simulink model "TosAeb"  (R2021a)
settings: Solver=FixedStepDiscrete  StartTime=0.0  StopTime=inf  FixedStep=0.01  RelTol=1e-3
undefined symbols (not defined in the file or in its folder): ACCModSts, AccSts1, AEB_ObjectMovingOrientation, AEB_ObjectMovingState, CN_BTCActive, CN_CurrentGearR, CN_CurrentGearR_DHT, CN_PTCActive, CN_VDCActive, CN_VSC_EspOff, CN_VSC_FCW_AEB_Error, CN_VSC_FCW_AEB_NoDispay, CN_VSC_FCW_AEB_PerfmDgdtn, CN_VSC_FCW_AEB_TempUnAvlbl, CN_VSC_VehStandstill, CNaccelAvoidMax, CNaccelAvoidMin, CNbrakePedalPress, CNcomfortStrLeftAccelMax, CNcomfortStrLeftAccelMin, CNcomfortStrRightAccelMax, CNcomfortStrRightAccelMin, CNDrvDoorOpen, CNDrvModeEco, CNDrvModeNorm, CNDrvModeOther, CNDrvModeSport, CNDrvSBRreminder, CNNull, CNobjInvalidID, CNPassDoorOpen, CNPassSBRreminder, CNPEBSAccelNegInf, CNPEBSAccelNull, CNPEBSTimeInf, CNPEBSTimeNull, CNSTOPMAX, CNSTOPNULL, CNsubjWidth, CNSwtOFF, CNSwtON, CNTTCMAX, CNTTCNULL, CNVehConfigAEBCycNone, CNVehConfigAEBNone, CNVehConfigAEBPedNone, CNVehConfigFCWNone, CNVehConfigJANone, CollisionSeverity, FALSE, FCWSnvty, JACycObjCalcInfoDefaultParams, JAPedObjCalcInfoDefaultParams, JAVehObjCalcInfoDefaultParams, latCycObjCalcInfoDefaultParams, latPedObjCalcInfoDefaultParams, longObjCalcInfoDefaultParams, ModSts, movingStatus, ObjectType ... (+1112)
libraries: simulink x3031, AEBSFunctionLib x50, Ta_Library x33, AEBSStateMachineLib x23, Mylibrary x12, simulink_extras x7, ObjSelect_lib x3, dspstat3 x2
(part 4 of 102)

== system TosAeb/FcwAebJa_Step_sys/ALG_AEBSystem/ALG_AEBController/AEB_FuncCondCalcApp ==
  note: Version : 20240729
  ACCModSts : Inport  OutDataTypeStr=Enum: ACCModSts
  LCK_Active : Inport  Port=2  OutDataTypeStr=boolean
  subjInfo : Inport  Port=3  OutDataTypeStr=Bus: BusSubjInfo  BusOutputAsStruct=on
  vehCanInfo : Inport  Port=4  OutDataTypeStr=Bus: BusVehCanInfo  BusOutputAsStruct=on
  vehConfigInfo : Inport  Port=5  OutDataTypeStr=Bus: BusVehConfigInfo  BusOutputAsStruct=on
  vehStateCalcInfo : Inport  Port=6  OutDataTypeStr=Bus: BusVehStateCalcInfo  BusOutputAsStruct=on
  longObjCalcInfo : Inport  Port=7  OutDataTypeStr=Bus: BusLongObjCalcInfo  BusOutputAsStruct=on
  latPedTgtCalcInfo : Inport  Port=8  OutDataTypeStr=Bus: BusLatPedTgtCalcInfo  BusOutputAsStruct=on
  latCycObjCalcInfo : Inport  Port=9  OutDataTypeStr=Bus: BusLatCycTgtCalcInfo  BusOutputAsStruct=on
  JAPedTgtCalcInfo : Inport  Port=10  OutDataTypeStr=Bus: BusAEBJAPedTgtCalcInfo  BusOutputAsStruct=on
  JAVehTgtCalcInfo : Inport  Port=11  OutDataTypeStr=Bus: BusAEBJAVehTgtCalcInfo  BusOutputAsStruct=on
  JaCycTgtCalcInfo : Inport  Port=12  OutDataTypeStr=Bus: BusAEBJACycTgtCalcInfo
  AEBModStsFuncImpArb : Inport  Port=13  OutDataTypeStr=Bus: BusAEBModStsFuncImpArb  BusOutputAsStruct=on
  latFuncActiveStateTime : Inport  Port=14  OutDataTypeStr=Bus: BusLatFuncActiveStateTime  BusOutputAsStruct=on
  Bus Creator : BusCreator  Inputs=14
  Bus Creator2 : BusCreator  Inputs=6  OutDataTypeStr=Bus: BusFuncCondCalcInfo  NonVirtualBus=on
      settings: InheritFromInputs=off
  Constant : Constant  Value=2024072901  OutDataTypeStr=uint32
  CycFunctionCondCalc : Subsystem
  JaCycFunctionCondCalc : Subsystem
  JaPedFunctionCondCalc : Subsystem
  JaVehFunctionCondCalc : Subsystem
  LongFunctionCondCalc : Subsystem
  PedFunctionCondCalc : Subsystem
  Terminator : Terminator
  FuncCondCalcInfo : Outport  OutDataTypeStr=Bus: BusFuncCondCalcInfo  BusOutputAsStruct=on
  wires:
  subjInfo/1 -> Bus Creator/3  "subjInfo"
  JAVehTgtCalcInfo/1 -> Bus Creator/11  "AEBJAVehTgtCalcInfo"
  LCK_Active/1 -> Bus Creator/2  "LCK_Active"
  CycFunctionCondCalc/1(cycFuncSuppAborCond) -> Bus Creator2/3  "cycFuncSuppAborCond"
  latCycObjCalcInfo/1 -> Bus Creator/9  "latCycTgtCalcInfo"
  LongFunctionCondCalc/1(vehFuncSuppAborCond) -> Bus Creator2/1  "vehFuncSuppAborCond"
  Bus Creator/1 -> LongFunctionCondCalc/1(inputSignals), PedFunctionCondCalc/1(inputSignals), JaPedFunctionCondCalc/1(inputSignals), JaCycFunctionCondCalc/1(inputSignals), JaVehFunctionCondCalc/1(inputSignals), CycFunctionCondCalc/1(inputSignals)  "inputSignals"
  JAPedTgtCalcInfo/1 -> Bus Creator/10  "AEBJAPedTgtCalcInfo"
  PedFunctionCondCalc/1(pedFuncSuppAborCond) -> Bus Creator2/2  "pedFuncSuppAborCond"
  latFuncActiveStateTime/1 -> Bus Creator/14  "latFuncActiveStateTime"
  vehStateCalcInfo/1 -> Bus Creator/5  "vehStateCalcInfo"
  Constant/1 -> Terminator/1  "AEB_FUNC_VERSION"
  longObjCalcInfo/1 -> Bus Creator/7  "longObjCalcInfo"
  JaPedFunctionCondCalc/1(AEBJAPedFuncSuppAborCond) -> Bus Creator2/4  "JAPedFuncSuppAborCond"
  vehCanInfo/1 -> Bus Creator/4  "vehCanInfo"
  JaCycFunctionCondCalc/1(JaCycFuncSuppAborCond) -> Bus Creator2/6  "JACycFuncSuppAborCond"
  JaVehFunctionCondCalc/1(AEBJAVehFuncSuppAborCond) -> Bus Creator2/5  "JAVehFuncSuppAborCond"
  JaCycTgtCalcInfo/1 -> Bus Creator/12  "JaCycTgtCalcInfo"
  latPedTgtCalcInfo/1 -> Bus Creator/8  "latPedTgtCalcInfo"
  ACCModSts/1 -> Bus Creator/1  "ACCModSts"
  AEBModStsFuncImpArb/1 -> Bus Creator/13  "AEBModStsFuncImpArb"
  vehConfigInfo/1 -> Bus Creator/6  "vehConfigInfo"
  Bus Creator2/1 -> FuncCondCalcInfo/1

== system TosAeb/FcwAebJa_Step_sys/ALG_AEBSystem/ALG_AEBController/AEB_FuncCondCalcInfo_FUS ==
  longObjCalcInfo : Inport
  subjInfo : Inport  Port=2  OutDataTypeStr=Bus: BusSubjInfo
  FuncCondCalcInfo : Inport  Port=3
  Bus Creator : BusCreator  Inputs=3
  Bus Creator2 : BusCreator  Inputs=6  OutDataTypeStr=Bus: BusFuncCondCalcInfo  NonVirtualBus=on
      settings: InheritFromInputs=off
  CycFunctionCondCalc : Subsystem
  JaCycFunctionCondCalc : Subsystem
  JaPedFunctionCondCalc : Subsystem
  JaVehFunctionCondCalc : Subsystem
  LongFunctionCondCalc : Subsystem
  PedFunctionCondCalc : Subsystem
  FuncCondCalcInfo_FUS : Outport  OutDataTypeStr=Bus: BusFuncCondCalcInfo
  wires:
  CycFunctionCondCalc/1(cycFuncSuppAborCond) -> Bus Creator2/3  "cycFuncSuppAborCond"
  longObjCalcInfo/1 -> Bus Creator/1  "longObjCalcInfo"
  JaCycFunctionCondCalc/1(JaCycFuncSuppAborCond) -> Bus Creator2/6  "JACycFuncSuppAborCond"
  PedFunctionCondCalc/1(pedFuncSuppAborCond) -> Bus Creator2/2  "pedFuncSuppAborCond"
  Bus Creator2/1 -> FuncCondCalcInfo_FUS/1
  Bus Creator/1 -> CycFunctionCondCalc/1(inputSignals), JaPedFunctionCondCalc/1(inputSignals), JaCycFunctionCondCalc/1(inputSignals), JaVehFunctionCondCalc/1(inputSignals), PedFunctionCondCalc/1(inputSignals), LongFunctionCondCalc/1(inputSignals)
  FuncCondCalcInfo/1 -> Bus Creator/3  "FuncCondCalcInfo"
  LongFunctionCondCalc/1(vehFuncSuppAborCond) -> Bus Creator2/1  "vehFuncSuppAborCond"
  JaPedFunctionCondCalc/1(AEBJAPedFuncSuppAborCond) -> Bus Creator2/4  "JAPedFuncSuppAborCond"
  JaVehFunctionCondCalc/1(AEBJAVehFuncSuppAborCond) -> Bus Creator2/5  "JAVehFuncSuppAborCond"
  subjInfo/1 -> Bus Creator/2  "subjInfo"

== system TosAeb/FcwAebJa_Step_sys/ALG_AEBSystem/ALG_AEBController/AEB_FuncImpManagementApp ==
  note: Version : 20211028
  note: Atuo Emergency Barke System Function Implement Management
  vehCanInfo : Inport  OutDataTypeStr=Bus: BusVehCanInfo
  subjVel : Inport  Port=2
  subjLongAccel : Inport  Port=3
  VSC_VehStandstill : Inport  Port=4  OutDataTypeStr=boolean
  longObjCalcInfo : Inport  Port=5  OutDataTypeStr=Bus: BusLongObjCalcInfo
  latPedTgtCalcInfo : Inport  Port=6  OutDataTypeStr=Bus: BusLatPedTgtCalcInfo
  latCycObjCalcInfo : Inport  Port=7  OutDataTypeStr=Bus: BusLatCycTgtCalcInfo
  AEBModStsArb : Inport  Port=8  OutDataTypeStr=Bus: BusAEBModStsArb
  Bus Creator : BusCreator  Inputs=7
  Bus Selector : BusSelector  OutputSignals=FCTB_BrkgReq,FCTB_ABA_Req,FCTB_ABP_Req
  Bus Selector2 : BusSelector  OutputSignals=vehCanInfo.AEBAvailable,longObjCalcInfo.LongObjRawInfo.ObjectMovingState,longObjCalcInfo.LongCollisionAssess.accelAvoidLowLvlBrk,longObjCalcInfo.longObjAbsInfo.axAbsObj,vehCanInfo.AEBBAActive,vehCanInfo.AEBIBActive,vehCanInfo.VehicleStandstill,VSC_VehStandstill,vehCanInfo.ABPAvailable,vehCanInfo.ABAavailable,longObjCalcInfo.LongCollisionAssess.longCollisionSeverity,vehCanInfo.ABPActive,vehCanInfo.ABAactive,vehCanInfo.FCTB_ABA_Level,subjVel,vehCanInfo.AWBactive,longObjCalcInfo.LongObjRawInfo.vx,longObjCalcInfo,latPedTgtCalcInfo,vehCanInfo.FCTB_BrkgReqVal,subjLongAccel
  FuncBlockTimeCalc : Subsystem
  FuncImpArbCalc : Subsystem  TreatAsAtomicUnit=on
  FuncManeuArbCalc : Subsystem
  Signal Conversion1 : SignalConversion  ConversionOutput=Nonvirtual bus  OutDataTypeStr=Bus: BusAEBSFuncOutput  OverrideOpt=off
  Signal Conversion2 : SignalConversion  ConversionOutput=Nonvirtual bus  OutDataTypeStr=Bus: BusFuncManeuAborCond  OverrideOpt=off
  AEBSFuncOut : Outport  OutDataTypeStr=Bus: BusAEBSFuncOutput
  FuncBlockTime : Outport  Port=2  OutDataTypeStr=Bus: BusFuncBlockTime
  AEBModStsFuncImpArb : Outport  Port=3  OutDataTypeStr=Bus: BusAEBModStsFuncImpArb
  FuncImpSuppAbor : Outport  Port=4  OutDataTypeStr=Bus: BusFuncImpSuppAborCond
  FuncManeuAborCond : Outport  Port=5  OutDataTypeStr=Bus: BusFuncManeuAborCond
  wires:
  Signal Conversion1/1 -> AEBSFuncOut/1
  Bus Selector2/2 -> FuncManeuArbCalc/3(ObjectMovingState)  "<ObjectMovingState>"
  Bus Selector/2 -> FuncImpArbCalc/3(FCTB_ABAModStsArb)  "<FCTB_ABA_Req>"
  Bus Selector2/20 -> FuncManeuArbCalc/21(FCTB_BrkgReqVal)  "<FCTB_BrkgReqVal>"
  vehCanInfo/1 -> Bus Creator/1, Bus Selector/1  "vehCanInfo"
  FuncBlockTimeCalc/1(funcBlockTime) -> FuncBlockTime/1
  subjVel/1 -> Bus Creator/3  "subjVel"
  Bus Selector2/14 -> FuncManeuArbCalc/15(FCTB_ABA_Level)  "<FCTB_ABA_Level>"
  AEBModStsArb/1 -> FuncImpArbCalc/1(AEBModStsArb)
  subjLongAccel/1 -> Bus Creator/6  "subjLongAccel"
  Bus Selector2/10 -> FuncManeuArbCalc/11(ABAAvailable)  "<ABAavailable>"
  Bus Selector2/12 -> FuncManeuArbCalc/13(ABPActive)  "<ABPActive>"
  VSC_VehStandstill/1 -> Bus Creator/7  "VSC_VehStandstill"
  Bus Selector2/8 -> FuncManeuArbCalc/9(VSC_VehStandstill)  "<VSC_VehStandstill>"
  Bus Selector2/17 -> FuncManeuArbCalc/18(vx)  "<vx>"
  FuncManeuArbCalc/1(AEBSFuncOutput) -> Signal Conversion1/1
  [AEBModStsFuncImpArb] -> AEBModStsFuncImpArb/1
  Bus Selector2/5 -> FuncManeuArbCalc/6(AEBBA)  "<AEBBAActive>"
  FuncManeuArbCalc/2(funcManeuAborCond) -> Signal Conversion2/1
  Bus Selector2/7 -> FuncManeuArbCalc/8(VehicleStandstill)  "<VehicleStandstill>"
  Signal Conversion2/1 -> FuncManeuAborCond/1
  Bus Selector2/3 -> FuncManeuArbCalc/4(accelAvoid)  "<accelAvoidLowLvlBrk>"
  Bus Selector/3 -> FuncImpArbCalc/4(FCTB_PrefillModStsArb)  "<FCTB_ABP_Req>"
  Bus Selector2/9 -> FuncManeuArbCalc/10(ABPAvailable)  "<ABPAvailable>"
  Bus Selector2/4 -> FuncManeuArbCalc/5(axAbsObj)  "<axAbsObj>"
  Bus Selector2/15 -> FuncManeuArbCalc/16(subjVel)  "<subjVel>"
  [funcImpSuppAbor] -> FuncImpSuppAbor/1
  Bus Selector2/11 -> FuncManeuArbCalc/12(longCollisionSeverity)  "<longCollisionSeverity>"
  latCycObjCalcInfo/1 -> Bus Creator/5  "latCycObjCalcInfo"
  Bus Creator/1 -> Bus Selector2/1
  longObjCalcInfo/1 -> Bus Creator/2  "longObjCalcInfo"
  Bus Selector2/6 -> FuncManeuArbCalc/7(AEBIB)  "<AEBIBActive>"
  Bus Selector2/18 -> FuncManeuArbCalc/19(longObjCalcInfo)  "<longObjCalcInfo>"
  Bus Selector/1 -> FuncImpArbCalc/2(FCTBModStsArb)  "<FCTB_BrkgReq>"
  FuncImpArbCalc/2(FuncImpSuppCondAbor) -> [funcImpSuppAbor]
  Bus Selector2/1 -> FuncManeuArbCalc/2(AEBAvailable)  "<AEBAvailable>"
  Bus Selector2/13 -> FuncManeuArbCalc/14(ABAActive)  "<ABAactive>"
  latPedTgtCalcInfo/1 -> Bus Creator/4  "latPedTgtCalcInfo"
  Bus Selector2/16 -> FuncManeuArbCalc/17(AWBActive)  "<AWBactive>"
  Bus Selector2/19 -> FuncManeuArbCalc/20(latPedTgtCalcInfo)  "<latPedTgtCalcInfo>"
  FuncImpArbCalc/1(AEBModStsFuncImpArb) -> [AEBModStsFuncImpArb], FuncManeuArbCalc/1(AEBModStsFuncImpArb), FuncBlockTimeCalc/1(AEBModStsFuncImpArb)
  Bus Selector2/21 -> FuncManeuArbCalc/22(subjLongAccel)  "<subjLongAccel>"

== system TosAeb/FcwAebJa_Step_sys/ALG_AEBSystem/ALG_AEBController/AEB_JaObjCalcApp ==
  JaObjInfo : Inport  OutDataTypeStr=Bus: BusAEBJAObjBus  PortDimensions=32  BusOutputAsStruct=on
  subjInfo : Inport  Port=2  OutDataTypeStr=Bus: BusSubjInfo
  SteerWheelAngle_Deg : Inport  Port=3
  JAPedPrefillModSts : Inport  Port=4  OutDataTypeStr=Enum: ModSts
  JAPedAEBfullModSts : Inport  Port=5  OutDataTypeStr=Enum: ModSts
  JAVehPrefillModSts : Inport  Port=6  OutDataTypeStr=Enum: ModSts
  JAVehAEBfullModSts : Inport  Port=7  OutDataTypeStr=Enum: ModSts
  JaCycPrefillModSts : Inport  Port=8  OutDataTypeStr=Enum: ModSts
  JaCycEBfullModSts : Inport  Port=9  OutDataTypeStr=Enum: ModSts
  Bus Creator : BusCreator  Inputs=9
  Bus Selector : BusSelector  OutputSignals=JaObjInfo,subjInfo,SteerWheelAngle_Deg,JAPedPrefillModSts,JAPedAEBfullModSts
  Bus Selector1 : BusSelector  OutputSignals=JaObjInfo,subjInfo,SteerWheelAngle_Deg,JAVehPrefillModSts,JAVehAEBfullModSts
  Bus Selector2 : BusSelector  OutputSignals=JaObjInfo,subjInfo,SteerWheelAngle_Deg,JaCycPrefillModSts,JaCycEBfullModSts
  JAPedTgtInfoCalc : Subsystem
  JAVehTgtInfoCalc : Subsystem
  JaCycTgtInfoCalc : Subsystem
  JaPedTgtCalcInfo : Outport  OutDataTypeStr=Bus: BusAEBJAPedTgtCalcInfo
  JaVehTgtCalcInfo : Outport  Port=2  OutDataTypeStr=Bus: BusAEBJAVehTgtCalcInfo
  JaCycTgtCalcInfo : Outport  Port=3  OutDataTypeStr=Bus: BusAEBJACycTgtCalcInfo
  wires:
  Bus Selector1/3 -> JAVehTgtInfoCalc/3(SteerWheelAngle_Deg)  "<SteerWheelAngle_Deg>"
  Bus Selector1/4 -> JAVehTgtInfoCalc/4(JAVehPrefillModSts)  "<JAVehPrefillModSts>"
  Bus Selector/4 -> JAPedTgtInfoCalc/4(JAPedPrefillModSts)  "<JAPedPrefillModSts>"
  JaCycEBfullModSts/1 -> Bus Creator/9  "JaCycEBfullModSts"
  Bus Selector/2 -> JAPedTgtInfoCalc/2(subjInfo)  "<subjInfo>"
  Bus Selector2/1 -> JaCycTgtInfoCalc/1(JaObj)  "<JaObjInfo>"
  Bus Selector2/5 -> JaCycTgtInfoCalc/5(JaCycAEBfullModSts)  "<JaCycEBfullModSts>"
  Bus Selector/1 -> JAPedTgtInfoCalc/1(AEBJAObj)  "<JaObjInfo>"
  JAPedPrefillModSts/1 -> Bus Creator/4  "JAPedPrefillModSts"
  Bus Selector2/4 -> JaCycTgtInfoCalc/4(JaCycPrefillModSts)  "<JaCycPrefillModSts>"
  Bus Selector2/3 -> JaCycTgtInfoCalc/3(SteerWheelAngle_Deg)  "<SteerWheelAngle_Deg>"
  Bus Selector1/2 -> JAVehTgtInfoCalc/2(subjInfo)  "<subjInfo>"
  Bus Selector/3 -> JAPedTgtInfoCalc/3(SteerWheelAngle_Deg)  "<SteerWheelAngle_Deg>"
  Bus Selector1/5 -> JAVehTgtInfoCalc/5(JAVehAEBfullModSts)  "<JAVehAEBfullModSts>"
  JaCycPrefillModSts/1 -> Bus Creator/8  "JaCycPrefillModSts"
  subjInfo/1 -> Bus Creator/2  "subjInfo"
  Bus Selector/5 -> JAPedTgtInfoCalc/5(JAPedAEBfullModSts)  "<JAPedAEBfullModSts>"
  SteerWheelAngle_Deg/1 -> Bus Creator/3  "SteerWheelAngle_Deg"
  JAPedTgtInfoCalc/1(AEBJAPedTgtCalcInfo) -> JaPedTgtCalcInfo/1
  JAVehAEBfullModSts/1 -> Bus Creator/7  "JAVehAEBfullModSts"
  JaCycTgtInfoCalc/1(JaCycTgtCalcInfo) -> JaCycTgtCalcInfo/1
  JAPedAEBfullModSts/1 -> Bus Creator/5  "JAPedAEBfullModSts"
  Bus Selector1/1 -> JAVehTgtInfoCalc/1(AEBJAObj)  "<JaObjInfo>"
  JaObjInfo/1 -> Bus Creator/1  "JaObjInfo"
  Bus Selector2/2 -> JaCycTgtInfoCalc/2(subjInfo)  "<subjInfo>"
  JAVehPrefillModSts/1 -> Bus Creator/6  "JAVehPrefillModSts"
  Bus Creator/1 -> Bus Selector2/1, Bus Selector1/1, Bus Selector/1
  JAVehTgtInfoCalc/1(JAVehTgtCalcInfo) -> JaVehTgtCalcInfo/1

== system TosAeb/FcwAebJa_Step_sys/ALG_AEBSystem/ALG_AEBController/AEB_LatObjInfoCalcApp ==
  note: Version : 20240729
  note: 1. 更新LatPedTgt终选条件，增加搜索目标ID 20221011
  FcwHMISetting : Inport  OutDataTypeStr=Enum: FCWSnvty
  latObjInfo : Inport  Port=2  OutDataTypeStr=Bus: BusAEBLatObj  PortDimensions=10  BusOutputAsStruct=on
  subjInfo : Inport  Port=3  OutDataTypeStr=Bus: BusSubjInfo
  LatCycObjCalc : Subsystem
  LatPedObjCalc : Subsystem
  latPedTgtCalcInfo : Outport  OutDataTypeStr=Bus: BusLatPedTgtCalcInfo
  latPedCalcInfo : Outport  Port=2  OutDataTypeStr=Bus: BusLatPedObjCalcInfo
  latCycTgtCalcInfo : Outport  Port=3  OutDataTypeStr=Bus: BusLatCycTgtCalcInfo
  latCycCalcInfo : Outport  Port=4  OutDataTypeStr=Bus: BusLatCycObjCalcInfo
  wires:
  LatPedObjCalc/1(latPedTgtCalcInfo) -> latPedTgtCalcInfo/1
  LatPedObjCalc/2(latPedCalcInfo) -> latPedCalcInfo/1
  LatCycObjCalc/1(latCycTgtCalcInfo) -> latCycTgtCalcInfo/1
  latObjInfo/1 -> LatCycObjCalc/1(latCycObjInfo), LatPedObjCalc/1(latPedObjInfo)  "latPedObjInfo"
  FcwHMISetting/1 -> LatPedObjCalc/3(FcwHMISetting)
  subjInfo/1 -> LatCycObjCalc/2(subjInfo), LatPedObjCalc/2(subjInfo)  "subjInfo"
  LatCycObjCalc/2(latCycCalcInfo) -> latCycCalcInfo/1

== system TosAeb/FcwAebJa_Step_sys/ALG_AEBSystem/ALG_AEBController/AEB_LongTgtInfoCalc ==
  note: AEBS Longitude Function Object Information Calculation
  note: 预测避免碰撞减速度Warning与Brake参数阈值分开处理 20240729
  note: Version : 20240729
  longObjInfo : Inport  OutDataTypeStr=Bus: BusAEBLongObj
  subjInfo : Inport  Port=2  OutDataTypeStr=Bus: BusSubjInfo
  ACCState : Inport  Port=3  OutDataTypeStr=Enum: ACCModSts
  vehCanInfo : Inport  Port=4  OutDataTypeStr=Bus: BusVehCanInfo
  FcwHMISetting : Inport  Port=5  OutDataTypeStr=Enum: FCWSnvty
  Bus Selector5 : BusSelector  OutputSignals=ObjectID
  If : If  IfExpression=u1
  LongObjCalcInfoCalc : Subsystem
  Merge : Merge
  NoTarget : Subsystem  TreatAsAtomicUnit=on
  ObjISInvalid : Subsystem
  longObjCalcInfo : Outport  OutDataTypeStr=Bus: BusLongObjCalcInfo
  wires:
  vehCanInfo/1 -> LongObjCalcInfoCalc/4(vehCanInfo)
  FcwHMISetting/1 -> LongObjCalcInfoCalc/5(FcwHMISetting)
  Bus Selector5/1 -> ObjISInvalid/1(ObjectID)  "<ObjectID>"
  If/2 -> LongObjCalcInfoCalc/Action
  Merge/1 -> longObjCalcInfo/1
  subjInfo/1 -> LongObjCalcInfoCalc/2(subjInfo)
  ACCState/1 -> LongObjCalcInfoCalc/3(ACCState)
  NoTarget/1(longObjCalcInfo) -> Merge/1
  ObjISInvalid/1(ObjIsInvalidFlg) -> If/1
  If/1 -> NoTarget/Action
  LongObjCalcInfoCalc/1(longObjCalcInfo) -> Merge/2
  longObjInfo/1 -> LongObjCalcInfoCalc/1(longObjInfo), Bus Selector5/1

== system TosAeb/FcwAebJa_Step_sys/ALG_AEBSystem/ALG_AEBController/AEB_StateMachineCalcApp ==
  note: AEB State Machine App
  note: Version : 20210816
  AVHStsActv : Inport
  vehStateCalcInfo : Inport  Port=2  OutDataTypeStr=Bus: BusVehStateCalcInfo
  FuncCondCalcInfo : Inport  Port=3  OutDataTypeStr=Bus: BusFuncCondCalcInfo
  FuncImpSuppAborCond : Inport  Port=4  OutDataTypeStr=Bus: BusFuncImpSuppAborCond
  FuncManeuAborCond : Inport  Port=5  OutDataTypeStr=Bus: BusFuncManeuAborCond
  Bus Creator : BusCreator  Inputs=6
  Bus Creator1 : BusCreator  Inputs=5
  Bus Creator2 : BusCreator  Inputs=7
  Bus Creator3 : BusCreator  Inputs=8
  Bus Creator4 : BusCreator  Inputs=3  OutDataTypeStr=Bus: BusAEBModStsArb  NonVirtualBus=on
      settings: InheritFromInputs=off
  Bus Creator5 : BusCreator  Inputs=3  OutDataTypeStr=Bus: BusAEBModRawSts  NonVirtualBus=on
      settings: InheritFromInputs=off
  Bus Selector : BusSelector  OutputSignals=AVHStsActv,vehStateCalcInfo,FuncCondCalcInfo.PedFuncSuppAborCond,FuncCondCalcInfo.CycFuncSuppAborCond,FuncImpSuppAborCond,FuncManeuAborCond
  Bus Selector1 : BusSelector  OutputSignals=AVHStsActv,vehStateCalcInfo,FuncCondCalcInfo.VehFuncSuppAborCond,FuncImpSuppAborCond,FuncManeuAborCond
  Bus Selector2 : BusSelector  OutputSignals=AVHStsActv,vehStateCalcInfo,FuncCondCalcInfo.JAPedFuncSuppAborCond,FuncCondCalcInfo.JAVehFuncSuppAborCond,FuncCondCalcInfo.JACycFuncSuppAborCond,FuncImpSuppAborCond,FuncManeuAborCond
  JA_AEBStateMachine : Subsystem
  JaFctStsArb : Subsystem
  LatAEBStateMachine : Subsystem
  LatFctStsArb : Subsystem
  Unit Delay : UnitDelay  InitialCondition=FALSE  SampleTime=-1  HasFrameUpgradeWarning=on
  Unit Delay1 : UnitDelay  InitialCondition=FALSE  SampleTime=-1  HasFrameUpgradeWarning=on
  Unit Delay2 : UnitDelay  InitialCondition=FALSE  SampleTime=-1  HasFrameUpgradeWarning=on
  VehAEBStateMachine : Subsystem
  VehFCTModStsArb : Subsystem
  AEBModStsArb : Outport  OutDataTypeStr=Bus: BusAEBModStsArb
  latActiveStateTime : Outport  Port=2  OutDataTypeStr=Bus: BusLatFuncActiveStateTime
  AEBModRawSts : Outport  Port=3  OutDataTypeStr=Bus: BusAEBModRawSts
  wires:
  Bus Selector2/4 -> Bus Creator3/4  "<JAVehFuncSuppAborCond>"
  VehFCTModStsArb/1(vehAEBModStsArb) -> Bus Creator4/1  "vehAEBModStsArb"
  JA_AEBStateMachine/1(JA_AEBRawModSts) -> [JA_AEBRawModSts]
  Unit Delay2/1 -> Bus Creator3/8  "JA_FctStsArbSuppAborCond"
  Bus Selector1/2 -> Bus Creator/2  "<vehStateCalcInfo>"
  Bus Selector/3 -> Bus Creator2/3  "<PedFuncSuppAborCond>"
  Bus Selector/6 -> Bus Creator2/6  "<FuncManeuAborCond>"
  vehStateCalcInfo/1 -> Bus Creator1/2  "vehStateCalcInfo"
  LatFctStsArb/1(latAEBModStsArb) -> Bus Creator4/2  "latAEBModStsArb"
  JA_AEBStateMachine/2(JA_AEBModSts) -> JaFctStsArb/1(JA_AEBModSts)
  Bus Selector/4 -> Bus Creator2/4  "<CycFuncSuppAborCond>"
  Bus Creator3/1 -> JA_AEBStateMachine/1(InputSignal)
  Bus Creator4/1 -> AEBModStsArb/1
  Unit Delay1/1 -> Bus Creator2/7  "latFctStsArbSuppAborCond"
  Bus Selector2/5 -> Bus Creator3/5  "<JACycFuncSuppAborCond>"
  Bus Selector1/5 -> Bus Creator/5  "<FuncManeuAborCond>"
  Bus Selector2/7 -> Bus Creator3/7  "<FuncManeuAborCond>"
  Bus Creator/1 -> VehAEBStateMachine/1(InputSignal)
  LatAEBStateMachine/1(latAEBRawModSts) -> [latAEBRawModSts]
  Bus Creator5/1 -> AEBModRawSts/1
  LatAEBStateMachine/3(latActiveStateTime) -> [latActiveStateTime]  "latActiveStateTime"
  [JA_AEBRawModSts] -> Bus Creator5/3  "JA_AEBRawModSts"
  VehAEBStateMachine/2(vehAEBModSts) -> VehFCTModStsArb/1(vehAEBModSts)
  Bus Selector2/3 -> Bus Creator3/3  "<JAPedFuncSuppAborCond>"
  VehAEBStateMachine/1(vehRawModSts) -> [vehRawModSts]
  Bus Selector1/1 -> Bus Creator/1  "<AVHStsActv>"
  [latAEBRawModSts] -> Bus Creator5/2  "latAEBRawModSts"
  LatFctStsArb/2(latFctStsArbSuppAborCond) -> Unit Delay1/1
  [latActiveStateTime] -> latActiveStateTime/1  "latActiveStateTime"
  FuncCondCalcInfo/1 -> Bus Creator1/3  "FuncCondCalcInfo"
  JaFctStsArb/2(vehFctStsArbSuppAborCond) -> Unit Delay2/1
  LatAEBStateMachine/2(latAEBModSts) -> LatFctStsArb/1(latAEBModSts)
  VehFCTModStsArb/2(vehFctStsArbSuppAborCond) -> Unit Delay/1
  Bus Selector1/4 -> Bus Creator/4  "<FuncImpSuppAborCond>"
  [vehRawModSts] -> Bus Creator5/1  "vehRawModSts"
  Bus Selector2/2 -> Bus Creator3/2  "<vehStateCalcInfo>"
  FuncImpSuppAborCond/1 -> Bus Creator1/4  "FuncImpSuppAborCond"
  Bus Creator1/1 -> Bus Selector2/1, Bus Selector/1, Bus Selector1/1
  Bus Selector1/3 -> Bus Creator/3  "<VehFuncSuppAborCond>"
  AVHStsActv/1 -> Bus Creator1/1  "AVHStsActv"
  Bus Selector2/6 -> Bus Creator3/6  "<FuncImpSuppAborCond>"
  Bus Selector/1 -> Bus Creator2/1  "<AVHStsActv>"
  Bus Selector/2 -> Bus Creator2/2  "<vehStateCalcInfo>"
  FuncManeuAborCond/1 -> Bus Creator1/5  "FuncManeuAborCond"
  Bus Selector2/1 -> Bus Creator3/1  "<AVHStsActv>"
  Bus Selector/5 -> Bus Creator2/5  "<FuncImpSuppAborCond>"
  Bus Creator2/1 -> LatAEBStateMachine/1(InputSignal)
  JaFctStsArb/1(JA_AEBModStsArb) -> Bus Creator4/3  "JA_pedAEBModStsArb"
  Unit Delay/1 -> Bus Creator/6  "vehFctStsArbSuppAborCond"

== system TosAeb/FcwAebJa_Step_sys/ALG_AEBSystem/ALG_AEBController/AEB_VehicleStateCalcApp ==
  note: Auto Emergency Brake System Vehicle State Calculation
  note: Version : 20210723
  diagManageInfo : Inport  OutDataTypeStr=Bus: BusDiagManageInfo
  vehCanInfo : Inport  Port=2  OutDataTypeStr=Bus: BusVehCanInfo
  vehConfigInfo : Inport  Port=3  OutDataTypeStr=Bus: BusVehConfigInfo
  subjVel : Inport  Port=4
  funcBlockTime : Inport  Port=5  OutDataTypeStr=Bus: BusFuncBlockTime
  Bus Creator : BusCreator  Inputs=4
  Bus Creator1 : BusCreator  Inputs=5
  Bus Creator2 : BusCreator  Inputs=3  OutDataTypeStr=Bus: BusVSC_FCW_AEB_FctSts
      settings: InheritFromInputs=off
  Bus Creator3 : BusCreator  Inputs=4  OutDataTypeStr=Bus: BusAEBFuncSwtSts
      settings: InheritFromInputs=off
  Bus Creator4 : BusCreator  Inputs=2
  Bus Creator6 : BusCreator  Inputs=7  OutDataTypeStr=Bus: BusVehStateCalcInfo  NonVirtualBus=on
      settings: InheritFromInputs=off
  Bus Selector : BusSelector  OutputSignals=diaManageInfo.FCW_AEB_ErrorState,diaManageInfo.AEB_PEDP_ErrorState,diaManageInfo.AEB_JA_ErrorState,vehCanInfo.EspFuncOffSts,vehCanInfo.TrailerSts
  Bus Selector1 : BusSelector  OutputSignals=vehCanInfo.AEB_VehSwtReq,vehCanInfo.AEB_PedSwtReq,vehCanInfo.AEB_JASwtReq,vehCanInfo.FCW_SnvtySet
  Bus Selector2 : BusSelector  OutputSignals=vehCanInfo.VDCActive,vehCanInfo.BTCActive,vehCanInfo.PTCActive,vehCanInfo.CURRENT_GEAR,vehCanInfo.HCU_GearSts,vehCanInfo.EspFuncOffSts,vehCanInfo.DrivingModeDis,vehCanInfo.DriverDoorSts,vehCanInfo.PassengerDoorSts,vehCanInfo.DriverSBR,vehCanInfo.PassSBR,vehCanInfo.EngState,vehCanInfo.HCU_PowertrainSts,vehCanInfo.HCU_Start_StopSts,subjVel,vehCanInfo.TrailerSts
  Bus Selector3 : BusSelector  OutputSignals=vehCanInfo.FLWheelSpd,vehCanInfo.FRWheelSpd,vehCanInfo.RLWheelSpd,vehCanInfo.RRWheelSpd,vehCanInfo.VehicleStandstill
  CycSuppAborCondtionCalc : Subsystem  TreatAsAtomicUnit=on
  FuncErrorStateJudge : Subsystem  TreatAsAtomicUnit=on
  FuncSwtStateJudge : Subsystem  TreatAsAtomicUnit=on
  JaSuppAborCondtionCalc : Subsystem  TreatAsAtomicUnit=on
  PedSuppAborCondtionCalc : Subsystem  TreatAsAtomicUnit=on
  VehMovingStateJudge : Subsystem  TreatAsAtomicUnit=on
  VehSuppAborCondtionCalc : Subsystem  TreatAsAtomicUnit=on
  vehStandStillCalc : Subsystem  TreatAsAtomicUnit=on
  vehStateCalcInfo : Outport  OutDataTypeStr=Bus: BusVehStateCalcInfo
  FCWSnvtyALG : Outport  Port=2
  wires:
  VehMovingStateJudge/1(vehUnstableStateFlg) -> Bus Creator4/1  "vehUnstableSts"
  Bus Creator3/1 -> Bus Creator/3, [VSC_AEBFuncSwtSts]  "VSC_AEBFuncSwtSts"
  Bus Selector2/14 -> VehMovingStateJudge/14(HCU_Start_StopSts)  "<HCU_Start_StopSts>"
  Bus Selector1/3 -> FuncSwtStateJudge/3(AEBJASwtReq)  "<AEB_JASwtReq>"
  subjVel/1 -> Bus Creator1/4  "subjVel"
  Bus Creator1/1 -> Bus Selector/1, Bus Selector1/1, Bus Selector2/1, Bus Selector3/1, Bus Creator/1  "vehStateCalcSignalInput"
  Bus Creator/1 -> VehSuppAborCondtionCalc/1(InputSignal), PedSuppAborCondtionCalc/1(InputSignal), JaSuppAborCondtionCalc/1(InputSignals), CycSuppAborCondtionCalc/1(InputSignal)
  JaSuppAborCondtionCalc/1(VSC_JAFuncSuppAborCond) -> Bus Creator6/4  "VSC_JAFuncSuppAborCond"
  PedSuppAborCondtionCalc/1(VSCPedFuncSuppAborCond) -> Bus Creator6/2  "VSCPedFuncSuppAborCond"
  Bus Selector2/9 -> VehMovingStateJudge/9(PassengerDoorSts)  "<PassengerDoorSts>"
  Bus Selector2/7 -> VehMovingStateJudge/7(DrivingModeDis)  "<DrivingModeDis>"
  diagManageInfo/1 -> Bus Creator1/1  "diaManageInfo"
  FuncErrorStateJudge/3(FCW_AEB_JAFctSts) -> Bus Creator2/3  "FCW_AEB_JAFctSts"
  Bus Creator2/1 -> [VSC_FCW_AEB_FctSts], Bus Creator/2  "VSC_FCW_AEB_FctSts"
  Bus Selector2/2 -> VehMovingStateJudge/2(BTCActive)  "<BTCActive>"
  Bus Selector2/4 -> VehMovingStateJudge/4(CURRENT_GEAR)  "<CURRENT_GEAR>"
  Bus Selector2/1 -> VehMovingStateJudge/1(VDCActive)  "<VDCActive>"
  Bus Selector3/3 -> vehStandStillCalc/3(RLWheelSpd)  "<RLWheelSpd>"
  FuncErrorStateJudge/1(FCW_AEB_VehFctSts) -> Bus Creator2/1  "FCW_AEB_VehFctSts"
  Bus Selector1/4 -> FuncSwtStateJudge/4(FCWSnvtySet)  "<FCW_SnvtySet>"
  Bus Selector/4 -> FuncErrorStateJudge/4(EspFuncOffSts)  "<EspFuncOffSts>"
  [VSC_FCW_AEB_FctSts] -> Bus Creator6/5  "VSC_FCW_AEB_FctSts"
  Bus Selector3/4 -> vehStandStillCalc/4(RRWheelSpd)  "<RRWheelSpd>"
  vehStandStillCalc/1(VSCVehStandStill) -> Bus Creator6/7  "VSCVehStandStill"
  FuncSwtStateJudge/2(AEB_PedResp) -> Bus Creator3/2  "AEB_PedResp"
  FuncSwtStateJudge/4(FCWSnvty) -> Bus Creator3/4  "FCWSnvty"
  Bus Creator4/1 -> Bus Creator/4  "vehMovingSts"
  VehMovingStateJudge/2(vehAssociatedSysStsSuppFlg) -> Bus Creator4/2  "vehAssociatedSysStsSuppFlg"
  funcBlockTime/1 -> Bus Creator1/5  "blockTime"
  Bus Selector2/15 -> VehMovingStateJudge/15(subjVel)  "<subjVel>"
  Bus Selector3/2 -> vehStandStillCalc/2(FRWheelSpd)  "<FRWheelSpd>"
  Bus Selector3/5 -> vehStandStillCalc/5(vehicleStandstill)  "<VehicleStandstill>"
  FuncErrorStateJudge/2(FCW_AEB_PedFctSts) -> Bus Creator2/2  "FCW_AEB_PedFctSts"
  Bus Selector2/13 -> VehMovingStateJudge/13(HCU_PowertrainSts)  "<HCU_PowertrainSts>"
  Bus Selector1/1 -> FuncSwtStateJudge/1(AEBVehSwtReq)  "<AEB_VehSwtReq>"
  FuncSwtStateJudge/3(AEB_JAResp) -> Bus Creator3/3  "AEB_JAResp"
  Bus Selector2/6 -> VehMovingStateJudge/6(EspFuncOffSts)  "<EspFuncOffSts>"
  Bus Selector2/10 -> VehMovingStateJudge/10(DriverSBR)  "<DriverSBR>"
  Bus Selector/1 -> FuncErrorStateJudge/1(FCW_AEB_ErrorState)  "<FCW_AEB_ErrorState>"
  FuncSwtStateJudge/1(AEB_VehResp) -> Bus Creator3/1  "AEB_VehResp"
  CycSuppAborCondtionCalc/1(VSCCycFuncSuppAborCond) -> Bus Creator6/3  "VSCCycFuncSuppAborCond"
  Bus Selector/3 -> FuncErrorStateJudge/3(AEB_JA_ErrorState)  "<AEB_JA_ErrorState>"
  VehSuppAborCondtionCalc/1(VSCVehSuppAborCond) -> Bus Creator6/1  "VSCVehSuppAborCond"
  Bus Creator6/1 -> vehStateCalcInfo/1
  Bus Selector2/3 -> VehMovingStateJudge/3(PTCActive)  "<PTCActive>"
  Bus Selector2/11 -> VehMovingStateJudge/11(PassSBR)  "<PassSBR>"
  Bus Selector1/2 -> FuncSwtStateJudge/2(AEBPedSwtReq)  "<AEB_PedSwtReq>"
  vehCanInfo/1 -> Bus Creator1/2  "vehCanInfo"
  Bus Selector2/16 -> VehMovingStateJudge/16(TrailerSts)  "<TrailerSts>"
  Bus Selector/5 -> FuncErrorStateJudge/5(TrailerSts)  "<TrailerSts>"
  Bus Selector2/5 -> VehMovingStateJudge/5(HCU_GearSts)  "<HCU_GearSts>"
  Bus Selector3/1 -> vehStandStillCalc/1(FLWheelSpd)  "<FLWheelSpd>"
  Bus Selector/2 -> FuncErrorStateJudge/2(AEB_Ped_ErrorState)  "<AEB_PEDP_ErrorState>"
  Bus Selector2/8 -> VehMovingStateJudge/8(DriverDoorSts)  "<DriverDoorSts>"
  Bus Selector2/12 -> VehMovingStateJudge/12(EngState)  "<EngState>"
  [VSC_AEBFuncSwtSts] -> Bus Creator6/6  "VSC_AEBFuncSwtSts"
  vehConfigInfo/1 -> Bus Creator1/3  "vehConfigInfo"
  FuncSwtStateJudge/5(FCWSnvtyALG) -> FCWSnvtyALG/1

== system TosAeb/FcwAebJa_Step_sys/ALG_AEBSystem/ALG_AEBInput/ADASStateMap ==
  note: LCK_Off(0)
      | LCK_Idle(1)
      | LCK_Ready(2)
      | LCK_Active(3)
      | LCK_Error(7)
  Compare To Constant : simulink/Logic and Bit Operations/Compare To Constant  relop===  const=uint8(3)  OutDataTypeStr=boolean
  Constant : Constant  Value=0  OutDataTypeStr=uint8
  Constant1 : Constant  Value=0  OutDataTypeStr=uint8
  Data Type Conversion : DataTypeConversion
  Data Type Conversion1 : DataTypeConversion
  ACCState : Outport
  LCK_Active : Outport  Port=2
  wires:
  Data Type Conversion/1 -> ACCState/1
  Compare To Constant/1 -> LCK_Active/1
  Data Type Conversion1/1 -> Compare To Constant/1
  Constant/1 -> Data Type Conversion/1  "ACCState"
  Constant1/1 -> Data Type Conversion1/1  "LCKState"

== system TosAeb/FcwAebJa_Step_sys/ALG_AEBSystem/ALG_AEBInput/DiaManageInfoMap ==
  Bus Creator : BusCreator  Inputs=3  OutDataTypeStr=Bus: BusDiagManageInfo
      settings: InheritFromInputs=off
  Compare To Constant : simulink/Logic and Bit Operations/Compare To Constant  relop===  const=uint8(1)  OutDataTypeStr=boolean
  Compare To Constant1 : simulink/Logic and Bit Operations/Compare To Constant  relop===  const=uint8(1)  OutDataTypeStr=boolean
  Compare To Constant2 : simulink/Logic and Bit Operations/Compare To Constant  relop===  const=uint8(1)  OutDataTypeStr=boolean
  Constant : Constant  Value=0  OutDataTypeStr=uint8
  Constant1 : Constant  Value=0  OutDataTypeStr=uint8
  Constant2 : Constant  Value=0  OutDataTypeStr=uint8
  Data Type Conversion : DataTypeConversion  OutDataTypeStr=boolean
  Data Type Conversion1 : DataTypeConversion  OutDataTypeStr=boolean
  Data Type Conversion2 : DataTypeConversion  OutDataTypeStr=boolean
  diaManageInfo : Outport
  wires:
  Data Type Conversion/1 -> Bus Creator/1
  Data Type Conversion1/1 -> Bus Creator/2
  Data Type Conversion2/1 -> Bus Creator/3
  Bus Creator/1 -> diaManageInfo/1
  Compare To Constant/1 -> Data Type Conversion/1
  Compare To Constant1/1 -> Data Type Conversion1/1
  Compare To Constant2/1 -> Data Type Conversion2/1
  Constant/1 -> Compare To Constant/1  "AEB_Error"
  Constant1/1 -> Compare To Constant1/1  "AEB_Ped_Error"
  Constant2/1 -> Compare To Constant2/1  "AEB_JA_Error"

== system TosAeb/FcwAebJa_Step_sys/ALG_AEBSystem/ALG_AEBInput/JaObjInfoMap ==
  TOS_AEB_JAOBJ : Inport  OutDataTypeStr=Bus: Object_Struct  Partition=on  PartitionDimension=1  PartitionWidth=1  PartitionOffset=0
  For Each : ForEach  DisableCoverage=on
  JaObjInfoMap : Subsystem
  JaObjInfo : Outport  OutDataTypeStr=Bus: BusAEBJAObjBus  ConcatenationDimension=1
  wires:
  JaObjInfoMap/1(JaObjInfo) -> JaObjInfo/1
  TOS_AEB_JAOBJ/1 -> JaObjInfoMap/1(TOS_AEB_JAOBJ)

== system TosAeb/FcwAebJa_Step_sys/ALG_AEBSystem/ALG_AEBInput/LatObjInfoMap ==
  TOS_AEB_LATOBJ : Inport  OutDataTypeStr=Bus: Object_Struct  Partition=on  PartitionDimension=1  PartitionWidth=1  PartitionOffset=0
  For Each : ForEach  DisableCoverage=on
  latObjInfoMap : Subsystem
  latObjInfo : Outport  OutDataTypeStr=Bus: BusAEBLatObj  ConcatenationDimension=1
  wires:
  latObjInfoMap/1(latObjInfo) -> latObjInfo/1
  TOS_AEB_LATOBJ/1 -> latObjInfoMap/1(TOS_AEB_LATOBJ)

== system TosAeb/FcwAebJa_Step_sys/ALG_AEBSystem/ALG_AEBInput/LongObjInfoMap ==
  TOS_AEB_LONOBJ : Inport  OutDataTypeStr=Bus: Object_Struct
  Bus Creator : BusCreator  Inputs=56  OutDataTypeStr=Bus: BusAEBLongObj  NonVirtualBus=on
      settings: InheritFromInputs=off
  Bus Selector1 : BusSelector  OutputSignals=objID,objType,objLength,objWidth,objHeight,azimuthAngle,yawAngle,movingStatus,movingDirection,dxCenter,dyCenter,vxRel,vyRel,axRel,ayRel,dxCenterStd,dyCenterStd,vxRelStd,vyRelStd,axRelStd,ayRelStd,objLengthStd,objWidthStd,yawAngleStd,objExitProb,objID,objType,objLatDist2LaneCenter,objLatDist2EgoCenter,movingStatus,movingDirection,dxCenter,dyCenter,vxRel,vyRel,objID,objType,movingStatus,movingDirection,dxCenter,dyCenter,vxRel,vyRel
  Data Type Conversion : DataTypeConversion  OutDataTypeStr=Enum: AEB_ObjectMovingOrientation
  Data Type Conversion1 : DataTypeConversion  OutDataTypeStr=Enum: AEB_ObjectMovingState
  Data Type Conversion10 : DataTypeConversion  OutDataTypeStr=uint16
  Data Type Conversion{11,41..42,46,51..52,54} (7 alike) : DataTypeConversion  OutDataTypeStr=uint8
  Data Type Conversion{2..7,12..36,38..40,43..45,48..50,53} (41 alike) : DataTypeConversion  OutDataTypeStr=single
  Data Type Conversion37 : DataTypeConversion  OutDataTypeStr=boolean
  Data Type Conversion47 : DataTypeConversion  OutDataTypeStr=uint16
  Data Type Conversion55 : DataTypeConversion  OutDataTypeStr=uint16
  Data Type Conversion8 : DataTypeConversion  OutDataTypeStr=boolean
  Data Type Conversion9 : DataTypeConversion  OutDataTypeStr=Enum: ObjectTypeCopy
  FusionType : Constant  OutDataTypeStr=single
  MovingStatus_Subsystem : Subsystem
  ObjType_Subsystem : Subsystem
  ObjectTypeCameraConfirmed : Constant  OutDataTypeStr=single
  Subsystem : Subsystem
  Subsystem1 : Subsystem
  dxFirstDetection : Constant  OutDataTypeStr=single
  dyFirstDetection : Constant  OutDataTypeStr=single
  dz : Constant  OutDataTypeStr=single
  objUpdate : Constant  Value=0  OutDataTypeStr=single
  objectIsObstacleProb : Constant  OutDataTypeStr=single
  objectLifeCycle : Constant  Value=0  OutDataTypeStr=single
  objectMeasurementStatus : Constant  Value=0  OutDataTypeStr=single
  objectMovingOrientationProb : Constant  OutDataTypeStr=single
  objectMovingStateProb : Constant  OutDataTypeStr=single
  objectRCS : Constant  OutDataTypeStr=single
  objectTypeProb : Constant  OutDataTypeStr=single
  longObjInfo : Outport  OutDataTypeStr=Bus: BusAEBLongObj
  wires:
  ObjType_Subsystem/1(ObjType) -> Data Type Conversion9/1  "ObjectType"
  Data Type Conversion30/1 -> Bus Creator/38  "dyObject2Center"
  objUpdate/1 -> Data Type Conversion37/1
  Data Type Conversion37/1 -> Bus Creator/40  "ObjUpdate"
  Bus Selector1/26 -> Data Type Conversion47/1  "<objID>"
  Bus Selector1/2 -> ObjType_Subsystem/1(Alg_ObjType)  "<objType>"
  objectIsObstacleProb/1 -> Data Type Conversion43/1
  Bus Selector1/29 -> Data Type Conversion31/1  "<objLatDist2EgoCenter>"
  ObjectTypeCameraConfirmed/1 -> Data Type Conversion8/1
  Bus Selector1/28 -> Data Type Conversion30/1  "<objLatDist2LaneCenter>"
  objectLifeCycle/1 -> Data Type Conversion34/1
  Bus Selector1/40 -> Data Type Conversion50/1  "<dxCenter>"
  Data Type Conversion40/1 -> Bus Creator/45  "FR_Dx"
  objectMovingOrientationProb/1 -> Data Type Conversion32/1
  objectMeasurementStatus/1 -> Data Type Conversion35/1
  Bus Selector1/6 -> Data Type Conversion3/1  "<azimuthAngle>"
  objectRCS/1 -> Data Type Conversion36/1
  Bus Selector1/41 -> Data Type Conversion48/1  "<dyCenter>"
  objectMovingStateProb/1 -> Data Type Conversion33/1
  Subsystem1/1(FC_ObjectTypeFilt) -> Bus Creator/50  "FC_ObjectType"
  dxFirstDetection/1 -> Data Type Conversion7/1
  objectTypeProb/1 -> Data Type Conversion24/1
  Data Type Conversion39/1 -> Bus Creator/47  "FR_Vx"
  Data Type Conversion42/1 -> Subsystem/2(FR_ObjectMovingState)  "FR_ObjectMovingState"
  dz/1 -> Data Type Conversion18/1
  Data Type Conversion49/1 -> Bus Creator/55  "FC_Vx"
  dyFirstDetection/1 -> Data Type Conversion6/1
  Bus Selector1/32 -> Data Type Conversion40/1  "<dxCenter>"
  Data Type Conversion38/1 -> Bus Creator/46  "FR_Dy"
  FusionType/1 -> Data Type Conversion11/1
  Data Type Conversion23/1 -> Bus Creator/23  "vxVariance"
  Data Type Conversion41/1 -> Subsystem/3(FR_ObjectMovingOrientation)  "FR_ObjectMovingOrientation"
  Data Type Conversion48/1 -> Bus Creator/54  "FC_Dy"
  TOS_AEB_LONOBJ/1 -> Bus Selector1/1
  Bus Selector1/36 -> Data Type Conversion55/1  "<objID>"
  Data Type Conversion54/1 -> Subsystem1/1(FC_ObjectType)  "FC_ObjectType"
  Data Type Conversion50/1 -> Bus Creator/53  "FC_Dx"
  Bus Selector1/34 -> Data Type Conversion39/1  "<vxRel>"
  Data Type Conversion47/1 -> Bus Creator/41  "FR_ObjectID"
  Data Type Conversion52/1 -> Subsystem1/2(FC_ObjectMovingState)  "FC_ObjectMovingState"
  Data Type Conversion51/1 -> Subsystem1/3(FC_ObjectMovingOrientation)  "FC_ObjectMovingOrientation"
  Bus Selector1/35 -> Data Type Conversion45/1  "<vyRel>"
  Data Type Conversion55/1 -> Bus Creator/49  "FC_ObjectID"
  Subsystem/1(FR_ObjectTypeFilt) -> Bus Creator/42  "FR_ObjectType"
  Subsystem1/3(FC_ObjectMovingOrientationFilt) -> Bus Creator/52  "FC_ObjectMovingOrientation"
  Data Type Conversion46/1 -> Subsystem/1(FR_ObjectType)  "FR_ObjectType"
  Subsystem1/2(FC_ObjectMovingStateFilt) -> Bus Creator/51  "FC_ObjectMovingState"
  Data Type Conversion31/1 -> Bus Creator/39  "dyEgo2Center"
  Bus Selector1/39 -> Data Type Conversion51/1  "<movingDirection>"
  Subsystem/2(FR_ObjectMovingStateFilt) -> Bus Creator/43  "FR_ObjectMovingState"
  Subsystem/3(FR_ObjectMovingOrientationFilt) -> Bus Creator/44  "FR_ObjectMovingOrientation"
  Bus Selector1/30 -> Data Type Conversion42/1  "<movingStatus>"
  Bus Selector1/43 -> Data Type Conversion53/1  "<vyRel>"
  Data Type Conversion45/1 -> Bus Creator/48  "FR_Vy"
  Data Type Conversion53/1 -> Bus Creator/56  "FC_Vy"
  Bus Selector1/33 -> Data Type Conversion38/1  "<dyCenter>"
  Bus Selector1/38 -> Data Type Conversion52/1  "<movingStatus>"
  Bus Selector1/42 -> Data Type Conversion49/1  "<vxRel>"
  Bus Selector1/27 -> Data Type Conversion46/1  "<objType>"
  Bus Selector1/31 -> Data Type Conversion41/1  "<movingDirection>"
  Bus Selector1/37 -> Data Type Conversion54/1  "<objType>"
  Bus Selector1/12 -> Data Type Conversion17/1  "<vxRel>"
  Data Type Conversion15/1 -> Bus Creator/5  "ObjectLength"
  Bus Selector1/15 -> Data Type Conversion21/1  "<ayRel>"
  Data Type Conversion17/1 -> Bus Creator/17  "vx"
  Data Type Conversion25/1 -> Bus Creator/29  "ObjectYawAngleVar"
  Data Type Conversion43/1 -> Bus Creator/34  "ObjectIsObstacleProb"
  Data Type Conversion33/1 -> Bus Creator/31  "ObjectMovingStateProb"
  Data Type Conversion27/1 -> Bus Creator/27  "ObjectLengthVar"
  Data Type Conversion18/1 -> Bus Creator/16  "dz"
  Bus Selector1/21 -> Data Type Conversion12/1  "<ayRelStd>"
  Data Type Conversion12/1 -> Bus Creator/26  "ayVariance"
  Data Type Conversion26/1 -> Bus Creator/28  "ObjectWidthVar"
  Bus Selector1/13 -> Data Type Conversion29/1  "<vyRel>"
  Data Type Conversion7/1 -> Bus Creator/12  "dxFisrtDetection"
  Bus Selector1/11 -> Data Type Conversion4/1  "<dyCenter>"
  Data Type Conversion32/1 -> Bus Creator/32  "ObjectMovingOrientationProb"
  Data Type Conversion34/1 -> Bus Creator/37  "ObjectLifeCycle"
  Bus Selector1/10 -> Data Type Conversion5/1  "<dxCenter>"
  Bus Selector1/14 -> Data Type Conversion28/1  "<axRel>"
  Data Type Conversion21/1 -> Bus Creator/20  "ay"
  Data Type Conversion19/1 -> Bus Creator/22  "dyVariance"
  Data Type Conversion22/1 -> Bus Creator/24  "vyVariance"
  Data Type Conversion2/1 -> Bus Creator/9  "ObjectYawAngle"
  Data Type Conversion11/1 -> Bus Creator/1  "FusionType"
  Data Type Conversion20/1 -> Bus Creator/21  "dxVariance"
  Data Type Conversion/1 -> Bus Creator/11  "ObjectMovingOrientation"
  Data Type Conversion13/1 -> Bus Creator/7  "ObjectHight"
  Bus Selector1/17 -> Data Type Conversion19/1  "<dyCenterStd>"
  Data Type Conversion4/1 -> Bus Creator/15  "dy"
  Data Type Conversion9/1 -> Bus Creator/3  "ObjectType"
  Data Type Conversion10/1 -> Bus Creator/2  "ObjectID"
  Data Type Conversion3/1 -> Bus Creator/8  "ObjectAzimuthAngle"
  Bus Selector1/4 -> Data Type Conversion14/1  "<objWidth>"
  Data Type Conversion44/1 -> Bus Creator/33  "ObjectExistProb"
  Data Type Conversion35/1 -> Bus Creator/36  "ObjectMeasurementStatus"
  Data Type Conversion36/1 -> Bus Creator/35  "ObjectRCS"
  Bus Selector1/25 -> Data Type Conversion44/1  "<objExitProb>"
  Data Type Conversion8/1 -> Bus Creator/4  "ObjectTypeCameraConfirmed"
  Bus Selector1/1 -> Data Type Conversion10/1  "<objID>"
  Data Type Conversion14/1 -> Bus Creator/6  "ObjectWidth"
  Bus Selector1/22 -> Data Type Conversion27/1  "<objLengthStd>"
  Data Type Conversion16/1 -> Bus Creator/25  "axVariance"
  Bus Selector1/5 -> Data Type Conversion13/1  "<objHeight>"
  Data Type Conversion29/1 -> Bus Creator/18  "vy"
  Data Type Conversion6/1 -> Bus Creator/13  "dyFisrtDetection"
  Bus Selector1/19 -> Data Type Conversion22/1  "<vyRelStd>"
  Data Type Conversion28/1 -> Bus Creator/19  "ax"
  Bus Selector1/18 -> Data Type Conversion23/1  "<vxRelStd>"
  Bus Selector1/16 -> Data Type Conversion20/1  "<dxCenterStd>"
  Bus Selector1/3 -> Data Type Conversion15/1  "<objLength>"
  Bus Selector1/7 -> Data Type Conversion2/1  "<yawAngle>"
  Bus Selector1/20 -> Data Type Conversion16/1  "<axRelStd>"
  Data Type Conversion1/1 -> Bus Creator/10  "ObjectMovingState"
  Data Type Conversion24/1 -> Bus Creator/30  "ObjectTypeProb"
  Data Type Conversion5/1 -> Bus Creator/14  "dx"
  Bus Selector1/9 -> Data Type Conversion/1  "<movingDirection>"
  Bus Selector1/23 -> Data Type Conversion26/1  "<objWidthStd>"
  Bus Selector1/24 -> Data Type Conversion25/1  "<yawAngleStd>"
  Bus Creator/1 -> longObjInfo/1
  MovingStatus_Subsystem/1(MovingStatusOut) -> Data Type Conversion1/1
  Bus Selector1/8 -> MovingStatus_Subsystem/1(Alg_MovingStatus)  "<movingStatus>"
